simulink model "TosAeb"  (R2021a)
settings: Solver=FixedStepDiscrete  StartTime=0.0  StopTime=inf  FixedStep=0.01  RelTol=1e-3
undefined symbols (not defined in the file or in its folder): ACCModSts, AccSts1, AEB_ObjectMovingOrientation, AEB_ObjectMovingState, CN_BTCActive, CN_CurrentGearR, CN_CurrentGearR_DHT, CN_PTCActive, CN_VDCActive, CN_VSC_EspOff, CN_VSC_FCW_AEB_Error, CN_VSC_FCW_AEB_NoDispay, CN_VSC_FCW_AEB_PerfmDgdtn, CN_VSC_FCW_AEB_TempUnAvlbl, CN_VSC_VehStandstill, CNaccelAvoidMax, CNaccelAvoidMin, CNbrakePedalPress, CNcomfortStrLeftAccelMax, CNcomfortStrLeftAccelMin, CNcomfortStrRightAccelMax, CNcomfortStrRightAccelMin, CNDrvDoorOpen, CNDrvModeEco, CNDrvModeNorm, CNDrvModeOther, CNDrvModeSport, CNDrvSBRreminder, CNNull, CNobjInvalidID, CNPassDoorOpen, CNPassSBRreminder, CNPEBSAccelNegInf, CNPEBSAccelNull, CNPEBSTimeInf, CNPEBSTimeNull, CNSTOPMAX, CNSTOPNULL, CNsubjWidth, CNSwtOFF, CNSwtON, CNTTCMAX, CNTTCNULL, CNVehConfigAEBCycNone, CNVehConfigAEBNone, CNVehConfigAEBPedNone, CNVehConfigFCWNone, CNVehConfigJANone, CollisionSeverity, FALSE, FCWSnvty, JACycObjCalcInfoDefaultParams, JAPedObjCalcInfoDefaultParams, JAVehObjCalcInfoDefaultParams, latCycObjCalcInfoDefaultParams, latPedObjCalcInfoDefaultParams, longObjCalcInfoDefaultParams, ModSts, movingStatus, ObjectType ... (+1112)
libraries: simulink x3031, AEBSFunctionLib x50, Ta_Library x33, AEBSStateMachineLib x23, Mylibrary x12, simulink_extras x7, ObjSelect_lib x3, dspstat3 x2
(part 10 of 102)

== system TosAeb/FcwAebJa_Step_sys/ALG_AEBSystem/ALG_AEBController/AEB_VehicleStateCalcApp/VehSuppAborCondtionCalc ==
  InputSignal : Inport
  ABASuppAborConditonCalc : Subsystem
  AEBfullSuppAborConditonCalc : Subsystem
  AEBpartSuppAborConditonCalc : Subsystem
  AcuteWarningSuppAborConditonCalc : Subsystem
  Bus Creator : BusCreator  Inputs=16  OutDataTypeStr=Bus: BusVSCVehSuppAborCond
      settings: InheritFromInputs=off
  Bus Selector : BusSelector  OutputSignals=VSC_AEBFuncSwtSts.AEB_VehResp,VSC_FCW_AEB_FctSts.FCW_AEB_VehFctSts,vehMovingSts.vehUnstableSts,vehMovingSts.vehAssociatedSysStsSuppFlg,vehStateCalcSignalInput.vehConfigInfo.VehicleConfigAEB,vehStateCalcSignalInput.blockTime.blockTimeBrakeReady
  Bus Selector1 : BusSelector  OutputSignals=VSC_AEBFuncSwtSts.AEB_VehResp,VSC_FCW_AEB_FctSts.FCW_AEB_VehFctSts,vehMovingSts.vehUnstableSts,vehMovingSts.vehAssociatedSysStsSuppFlg,vehStateCalcSignalInput.vehConfigInfo.VehicleConfigAEB,vehStateCalcSignalInput.blockTime.blockTimeBrakeReady
  Bus Selector2 : BusSelector  OutputSignals=VSC_AEBFuncSwtSts.AEB_VehResp,VSC_FCW_AEB_FctSts.FCW_AEB_VehFctSts,vehMovingSts.vehUnstableSts,vehMovingSts.vehAssociatedSysStsSuppFlg,vehStateCalcSignalInput.vehConfigInfo.VehicleConfigFCW,vehStateCalcSignalInput.blockTime.blockTimeWarning
  Bus Selector3 : BusSelector  OutputSignals=VSC_AEBFuncSwtSts.AEB_VehResp,VSC_FCW_AEB_FctSts.FCW_AEB_VehFctSts,vehMovingSts.vehUnstableSts,vehMovingSts.vehAssociatedSysStsSuppFlg,vehStateCalcSignalInput.vehConfigInfo.VehicleConfigFCW,vehStateCalcSignalInput.blockTime.blockTimeWarning
  Bus Selector4 : BusSelector  OutputSignals=VSC_AEBFuncSwtSts.AEB_VehResp,VSC_FCW_AEB_FctSts.FCW_AEB_VehFctSts,vehMovingSts.vehUnstableSts,vehMovingSts.vehAssociatedSysStsSuppFlg,vehStateCalcSignalInput.vehConfigInfo.VehicleConfigAEB,vehStateCalcSignalInput.blockTime.blockTimeBrake
  Bus Selector5 : BusSelector  OutputSignals=VSC_AEBFuncSwtSts.AEB_VehResp,VSC_FCW_AEB_FctSts.FCW_AEB_VehFctSts,vehMovingSts.vehUnstableSts,vehMovingSts.vehAssociatedSysStsSuppFlg,vehStateCalcSignalInput.vehConfigInfo.VehicleConfigFCW,vehStateCalcSignalInput.blockTime.blockTimeWarning
  Bus Selector6 : BusSelector  OutputSignals=VSC_AEBFuncSwtSts.AEB_VehResp,VSC_FCW_AEB_FctSts.FCW_AEB_VehFctSts,vehMovingSts.vehUnstableSts,vehMovingSts.vehAssociatedSysStsSuppFlg,vehStateCalcSignalInput.vehConfigInfo.VehicleConfigAEB,vehStateCalcSignalInput.blockTime.blockTimeBrake
  Bus Selector7 : BusSelector  OutputSignals=VSC_AEBFuncSwtSts.AEB_VehResp,VSC_FCW_AEB_FctSts.FCW_AEB_VehFctSts,vehMovingSts.vehUnstableSts,vehMovingSts.vehAssociatedSysStsSuppFlg,vehStateCalcSignalInput.vehConfigInfo.VehicleConfigAEB,vehStateCalcSignalInput.blockTime.blockTimeBrake
  EBASuppAborConditonCalc : Subsystem
  LatentWarningSuppAborConditonCalc : Subsystem
  PreWarningSuppAborConditonCalc : Subsystem
  PrefillSuppAborConditonCalc : Subsystem
  VSCVehSuppAborCond : Outport  OutDataTypeStr=Bus: BusVSCVehSuppAborCond
  wires:
  PreWarningSuppAborConditonCalc/1(VSCvehPreWarningSuppCond) -> Bus Creator/1  "VSCvehPreWarningSuppCond"
  PreWarningSuppAborConditonCalc/2(VSCvehPreWarningAborCond) -> Bus Creator/2  "VSCvehPreWarningAborCond"
  EBASuppAborConditonCalc/2(VSCvehEBAAborCond) -> Bus Creator/16  "VSCvehEBAAborCond"
  EBASuppAborConditonCalc/1(VSCvehEBASuppCond) -> Bus Creator/15  "VSCvehEBASuppCond"
  AcuteWarningSuppAborConditonCalc/1(VSCvehAcuteWarningSuppCond) -> Bus Creator/3  "VSCvehAcuteWarningSuppCond"
  AcuteWarningSuppAborConditonCalc/2(VSCvehAcuteWarningAborCond) -> Bus Creator/4  "VSCvehAcuteWarningAborCond"
  AEBfullSuppAborConditonCalc/2(VSCvehAEBfullAborCond) -> Bus Creator/14  "VSCvehAEBfullAborCond"
  AEBfullSuppAborConditonCalc/1(VSCvehAEBfullSuppCond) -> Bus Creator/13  "VSCvehAEBfullSuppCond"
  LatentWarningSuppAborConditonCalc/1(VSCvehLatentWarningSuppCond) -> Bus Creator/5  "VSCvehLatentWarningSuppCond"
  LatentWarningSuppAborConditonCalc/2(VSCvehLatentWarningAborCond) -> Bus Creator/6  "VSCvehLatentWarningAborCond"
  AEBpartSuppAborConditonCalc/2(VSCvehAEBpartAborCond) -> Bus Creator/12  "VSCvehAEBpartAborCond"
  AEBpartSuppAborConditonCalc/1(VSCvehAEBpartSuppCond) -> Bus Creator/11  "VSCvehAEBpartSuppCond"
  PrefillSuppAborConditonCalc/1(VSCvehPrefillSuppCond) -> Bus Creator/7  "VSCvehPrefillSuppCond"
  PrefillSuppAborConditonCalc/2(VSCvehPrefillAborCond) -> Bus Creator/8  "VSCvehPrefillAborCond"
  ABASuppAborConditonCalc/2(VSCvehABAAborCond) -> Bus Creator/10  "VSCvehABAAborCond"
  ABASuppAborConditonCalc/1(VSCvehABASuppCond) -> Bus Creator/9  "VSCvehABASuppCond"
  Bus Creator/1 -> VSCVehSuppAborCond/1
  Bus Selector7/6 -> EBASuppAborConditonCalc/6(blockTimeBrake)  "<blockTimeBrake>"
  Bus Selector7/5 -> EBASuppAborConditonCalc/5("vehConfigAEB ")  "<VehicleConfigAEB>"
  Bus Selector7/4 -> EBASuppAborConditonCalc/4(vehAssociatedSysStateSuppCond)  "<vehAssociatedSysStsSuppFlg>"
  Bus Selector7/3 -> EBASuppAborConditonCalc/3(vehUnstableStateFlg)  "<vehUnstableSts>"
  Bus Selector7/2 -> EBASuppAborConditonCalc/2(FCW_AEB_VehFctSts)  "<FCW_AEB_VehFctSts>"
  Bus Selector7/1 -> EBASuppAborConditonCalc/1(AEB_VehResp)  "<AEB_VehResp>"
  Bus Selector6/6 -> AEBpartSuppAborConditonCalc/6(blockTimeBrake)  "<blockTimeBrake>"
  Bus Selector6/5 -> AEBpartSuppAborConditonCalc/5("vehConfigAEB ")  "<VehicleConfigAEB>"
  Bus Selector6/4 -> AEBpartSuppAborConditonCalc/4(vehAssociatedSysStateSuppCond)  "<vehAssociatedSysStsSuppFlg>"
  Bus Selector6/3 -> AEBpartSuppAborConditonCalc/3(vehUnstableStateFlg)  "<vehUnstableSts>"
  Bus Selector6/2 -> AEBpartSuppAborConditonCalc/2(FCW_AEB_VehFctSts)  "<FCW_AEB_VehFctSts>"
  Bus Selector6/1 -> AEBpartSuppAborConditonCalc/1(AEB_VehResp)  "<AEB_VehResp>"
  InputSignal/1 -> Bus Selector2/1, Bus Selector5/1, Bus Selector/1, Bus Selector1/1, Bus Selector6/1, Bus Selector4/1, Bus Selector7/1, Bus Selector3/1
  Bus Selector4/6 -> AEBfullSuppAborConditonCalc/6(blockTimeBrake)  "<blockTimeBrake>"
  Bus Selector4/5 -> AEBfullSuppAborConditonCalc/5("vehConfigAEB ")  "<VehicleConfigAEB>"
  Bus Selector4/4 -> AEBfullSuppAborConditonCalc/4(vehAssociatedSysStateSuppCond)  "<vehAssociatedSysStsSuppFlg>"
  Bus Selector4/3 -> AEBfullSuppAborConditonCalc/3(vehUnstableStateFlg)  "<vehUnstableSts>"
  Bus Selector4/2 -> AEBfullSuppAborConditonCalc/2(FCW_AEB_VehFctSts)  "<FCW_AEB_VehFctSts>"
  Bus Selector4/1 -> AEBfullSuppAborConditonCalc/1(VSCvehAEB_VehResp)  "<AEB_VehResp>"
  Bus Selector5/6 -> LatentWarningSuppAborConditonCalc/6(blockTimeWarning)  "<blockTimeWarning>"
  Bus Selector5/5 -> LatentWarningSuppAborConditonCalc/5("vehConfigFCW ")  "<VehicleConfigFCW>"
  Bus Selector5/4 -> LatentWarningSuppAborConditonCalc/4(vehAssociatedSysStateSuppCond)  "<vehAssociatedSysStsSuppFlg>"
  Bus Selector5/3 -> LatentWarningSuppAborConditonCalc/3(vehUnstableStateFlg)  "<vehUnstableSts>"
  Bus Selector5/2 -> LatentWarningSuppAborConditonCalc/2(FCW_AEB_VehFctSts)  "<FCW_AEB_VehFctSts>"
  Bus Selector5/1 -> LatentWarningSuppAborConditonCalc/1(AEB_VehResp)  "<AEB_VehResp>"
  Bus Selector3/6 -> AcuteWarningSuppAborConditonCalc/6(blockTimeWarning)  "<blockTimeWarning>"
  Bus Selector3/5 -> AcuteWarningSuppAborConditonCalc/5("vehConfigFCW ")  "<VehicleConfigFCW>"
  Bus Selector3/4 -> AcuteWarningSuppAborConditonCalc/4(vehAssociatedSysStateSuppCond)  "<vehAssociatedSysStsSuppFlg>"
  Bus Selector3/3 -> AcuteWarningSuppAborConditonCalc/3(vehUnstableStateFlg)  "<vehUnstableSts>"
  Bus Selector3/2 -> AcuteWarningSuppAborConditonCalc/2(FCW_AEB_VehFctSts)  "<FCW_AEB_VehFctSts>"
  Bus Selector3/1 -> AcuteWarningSuppAborConditonCalc/1(AEB_VehResp)  "<AEB_VehResp>"
  Bus Selector2/6 -> PreWarningSuppAborConditonCalc/6(blockTimeWarning)  "<blockTimeWarning>"
  Bus Selector2/5 -> PreWarningSuppAborConditonCalc/5("vehConfigFCW ")  "<VehicleConfigFCW>"
  Bus Selector2/4 -> PreWarningSuppAborConditonCalc/4(vehAssociatedSysSuppStateFlg)  "<vehAssociatedSysStsSuppFlg>"
  Bus Selector2/3 -> PreWarningSuppAborConditonCalc/3(vehUnstableStateFlg)  "<vehUnstableSts>"
  Bus Selector2/2 -> PreWarningSuppAborConditonCalc/2(FCW_AEB_VehFctSts)  "<FCW_AEB_VehFctSts>"
  Bus Selector2/1 -> PreWarningSuppAborConditonCalc/1(AEB_VehResp)  "<AEB_VehResp>"
  Bus Selector1/6 -> ABASuppAborConditonCalc/6(blockTimeBrakeReady)  "<blockTimeBrakeReady>"
  Bus Selector1/5 -> ABASuppAborConditonCalc/5("vehConfigAEB ")  "<VehicleConfigAEB>"
  Bus Selector1/4 -> ABASuppAborConditonCalc/4(vehAssociatedSysStateSuppCond)  "<vehAssociatedSysStsSuppFlg>"
  Bus Selector1/3 -> ABASuppAborConditonCalc/3(vehUnstableStateFlg)  "<vehUnstableSts>"
  Bus Selector1/2 -> ABASuppAborConditonCalc/2(FCW_AEB_VehFctSts)  "<FCW_AEB_VehFctSts>"
  Bus Selector1/1 -> ABASuppAborConditonCalc/1(AEB_VehResp)  "<AEB_VehResp>"
  Bus Selector/6 -> PrefillSuppAborConditonCalc/6(blockTimeBrakeReady)  "<blockTimeBrakeReady>"
  Bus Selector/1 -> PrefillSuppAborConditonCalc/1(AEB_VehResp)  "<AEB_VehResp>"
  Bus Selector/5 -> PrefillSuppAborConditonCalc/5("vehConfigAEB ")  "<VehicleConfigAEB>"
  Bus Selector/2 -> PrefillSuppAborConditonCalc/2(FCW_AEB_VehFctSts)  "<FCW_AEB_VehFctSts>"
  Bus Selector/4 -> PrefillSuppAborConditonCalc/4(vehAssociatedSysStateSuppCond)  "<vehAssociatedSysStsSuppFlg>"
  Bus Selector/3 -> PrefillSuppAborConditonCalc/3(vehUnstableStateFlg)  "<vehUnstableSts>"

== system TosAeb/FcwAebJa_Step_sys/ALG_AEBSystem/ALG_AEBController/AEB_VehicleStateCalcApp/vehStandStillCalc ==
  note: FLWheelSpd + FRWheelSpd + RLWheelSpd + RRWheelSpd
      | subjWheelSpd = ---------------------------------------------------------------------------------------
      | 											4
  FLWheelSpd : Inport
  FRWheelSpd : Inport  Port=2
  RLWheelSpd : Inport  Port=3
  RRWheelSpd : Inport  Port=4
  vehicleStandstill : Inport  Port=5
  Add : Sum  Inputs=4
  Compare To Constant : simulink/Logic and Bit Operations/Compare To Constant  relop===  const=CN_VSC_VehStandstill  OutDataTypeStr=boolean
  Compare To Constant1 : simulink/Logic and Bit Operations/Compare To Constant  relop=<  const=P_AEB_vehStateCalc_VehWheelThres  OutDataTypeStr=boolean
  Gain : Gain  Gain=0.25
  Logical Operator : Logic  Operator=OR  AllPortsSameDT=off  OutDataTypeStr=boolean
  VSCVehStandStill : Outport
  wires:
  Logical Operator/1 -> VSCVehStandStill/1
  Compare To Constant/1 -> Logical Operator/2
  Compare To Constant1/1 -> Logical Operator/1
  vehicleStandstill/1 -> Compare To Constant/1
  Gain/1 -> Compare To Constant1/1  "subjWheelSpdAverage"
  Add/1 -> Gain/1
  RRWheelSpd/1 -> Add/4
  RLWheelSpd/1 -> Add/3
  FRWheelSpd/1 -> Add/2
  FLWheelSpd/1 -> Add/1

== system TosAeb/FcwAebJa_Step_sys/ALG_AEBSystem/ALG_AEBInput/JaObjInfoMap/JaObjInfoMap ==
  TOS_AEB_JAOBJ : Inport  OutDataTypeStr=Bus: Object_Struct
  Bus Creator : BusCreator  Inputs=54  OutDataTypeStr=Bus: BusAEBJAObjBus  NonVirtualBus=on
      settings: InheritFromInputs=off
  Bus Selector2 : BusSelector  OutputSignals=objID,objType,objLength,objWidth,objHeight,azimuthAngle,yawAngle,movingStatus,movingDirection,dxCenter,dyCenter,vxRel,vyRel,axRel,ayRel,dxCenterStd,dyCenterStd,vxRelStd,vyRelStd,axRelStd,ayRelStd,objLengthStd,objWidthStd,yawAngleStd,objExitProb,objID,objType,movingStatus,movingDirection,dxCenter,dyCenter,vxRel,vyRel,objID,objType,movingStatus,movingDirection,dxCenter,dyCenter,vxRel,vyRel
  Data Type Conversion{,2..8,11..30,32..36,38..46,48..54} (49 alike) : DataTypeConversion
  Data Type Conversion1 : DataTypeConversion  OutDataTypeStr=Enum: AEB_ObjectMovingState
  Data Type Conversion10 : DataTypeConversion  OutDataTypeStr=uint16
  Data Type Conversion31 : DataTypeConversion  OutDataTypeStr=Enum: ObjectTypeCopy
  Data Type Conversion47 : DataTypeConversion  OutDataTypeStr=uint16
  Data Type Conversion55 : DataTypeConversion  OutDataTypeStr=uint16
  FusionType : Constant  OutDataTypeStr=single
  MovingStatus_Subsystem : Subsystem
  ObjType_Subsystem : Subsystem
  ObjectTypeCameraConfirmed : Constant  OutDataTypeStr=single
  Subsystem : Subsystem
  Subsystem1 : Subsystem
  dxFirstDetection : Constant  OutDataTypeStr=single
  dyFirstDetection : Constant  OutDataTypeStr=single
  dz : Constant  OutDataTypeStr=single
  objUpdate : Constant  Value=0  OutDataTypeStr=single
  objectIsObstacleProb : Constant  OutDataTypeStr=single
  objectLifeCycle : Constant  Value=0  OutDataTypeStr=single
  objectMeasurementStatus : Constant  Value=0  OutDataTypeStr=single
  objectMovingOrientationProb : Constant  OutDataTypeStr=single
  objectMovingStateProb : Constant  OutDataTypeStr=single
  objectRCS : Constant  OutDataTypeStr=single
  objectTypeProb : Constant  OutDataTypeStr=single
  JaObjInfo : Outport  OutDataTypeStr=Bus: BusAEBJAObjBus
  wires:
  ObjType_Subsystem/1(ObjType) -> Data Type Conversion31/1  "ObjectType"
  objectMeasurementStatus/1 -> Data Type Conversion35/1
  Bus Selector2/17 -> Data Type Conversion19/1  "<dyCenterStd>"
  objectTypeProb/1 -> Data Type Conversion24/1
  dz/1 -> Data Type Conversion18/1
  Bus Selector2/21 -> Data Type Conversion12/1  "<ayRelStd>"
  Bus Selector2/13 -> Data Type Conversion29/1  "<vyRel>"
  Bus Selector2/25 -> Data Type Conversion44/1  "<objExitProb>"
  Bus Selector2/18 -> Data Type Conversion23/1  "<vxRelStd>"
  objectLifeCycle/1 -> Data Type Conversion34/1
  objectRCS/1 -> Data Type Conversion36/1
  dyFirstDetection/1 -> Data Type Conversion6/1
  Bus Selector2/10 -> Data Type Conversion5/1  "<dxCenter>"
  Bus Selector2/14 -> Data Type Conversion28/1  "<axRel>"
  Bus Selector2/22 -> Data Type Conversion27/1  "<objLengthStd>"
  Bus Selector2/7 -> Data Type Conversion2/1  "<yawAngle>"
  Bus Selector2/3 -> Data Type Conversion15/1  "<objLength>"
  Bus Selector2/4 -> Data Type Conversion14/1  "<objWidth>"
  Bus Selector2/32 -> Data Type Conversion39/1  "<vxRel>"
  Bus Selector2/28 -> Data Type Conversion42/1  "<movingStatus>"
  Bus Selector2/36 -> Data Type Conversion52/1  "<movingStatus>"
  Bus Selector2/40 -> Data Type Conversion49/1  "<vxRel>"
  Bus Selector2/33 -> Data Type Conversion45/1  "<vyRel>"
  Bus Selector2/41 -> Data Type Conversion53/1  "<vyRel>"
  Bus Selector2/37 -> Data Type Conversion51/1  "<movingDirection>"
  Bus Selector2/29 -> Data Type Conversion41/1  "<movingDirection>"
  objUpdate/1 -> Data Type Conversion30/1
  Bus Selector2/20 -> Data Type Conversion16/1  "<axRelStd>"
  objectMovingOrientationProb/1 -> Data Type Conversion32/1
  Bus Selector2/12 -> Data Type Conversion17/1  "<vxRel>"
  Bus Selector2/16 -> Data Type Conversion20/1  "<dxCenterStd>"
  Bus Selector2/24 -> Data Type Conversion25/1  "<yawAngleStd>"
  FusionType/1 -> Data Type Conversion11/1
  Bus Selector2/2 -> ObjType_Subsystem/1(Alg_ObjType)  "<objType>"
  Bus Selector2/6 -> Data Type Conversion3/1  "<azimuthAngle>"
  objectIsObstacleProb/1 -> Data Type Conversion43/1
  Bus Selector2/15 -> Data Type Conversion21/1  "<ayRel>"
  objectMovingStateProb/1 -> Data Type Conversion33/1
  Bus Selector2/11 -> Data Type Conversion4/1  "<dyCenter>"
  Bus Selector2/19 -> Data Type Conversion22/1  "<vyRelStd>"
  dxFirstDetection/1 -> Data Type Conversion7/1
  Bus Selector2/23 -> Data Type Conversion26/1  "<objWidthStd>"
  Bus Selector2/9 -> Data Type Conversion/1  "<movingDirection>"
  Bus Selector2/1 -> Data Type Conversion10/1  "<objID>"
  Bus Selector2/5 -> Data Type Conversion13/1  "<objHeight>"
  Bus Selector2/38 -> Data Type Conversion50/1  "<dxCenter>"
  Bus Selector2/34 -> Data Type Conversion55/1  "<objID>"
  Bus Selector2/30 -> Data Type Conversion40/1  "<dxCenter>"
  Bus Selector2/26 -> Data Type Conversion47/1  "<objID>"
  Bus Selector2/39 -> Data Type Conversion48/1  "<dyCenter>"
  Bus Selector2/31 -> Data Type Conversion38/1  "<dyCenter>"
  Bus Selector2/27 -> Data Type Conversion46/1  "<objType>"
  Bus Selector2/35 -> Data Type Conversion54/1  "<objType>"
  TOS_AEB_JAOBJ/1 -> Bus Selector2/1
  Data Type Conversion19/1 -> Bus Creator/22  "dyVariance"
  ObjectTypeCameraConfirmed/1 -> Data Type Conversion8/1
  Data Type Conversion47/1 -> Bus Creator/39  "FR_ObjectID"
  Data Type Conversion45/1 -> Bus Creator/46  "FR_Vy"
  Data Type Conversion55/1 -> Bus Creator/47  "FC_ObjectID"
  Data Type Conversion54/1 -> Subsystem1/1(FC_ObjectType)  "FC_ObjectType"
  Data Type Conversion38/1 -> Bus Creator/44  "FR_Dy"
  Data Type Conversion39/1 -> Bus Creator/45  "FR_Vx"
  Subsystem/1(FR_ObjectTypeFilt) -> Bus Creator/40  "FR_ObjectType"
  Data Type Conversion41/1 -> Subsystem/3(FR_ObjectMovingOrientation)  "FR_ObjectMovingOrientation"
  Subsystem1/3(FC_ObjectMovingOrientationFilt) -> Bus Creator/50  "FC_ObjectMovingOrientation"
  Subsystem1/2(FC_ObjectMovingStateFilt) -> Bus Creator/49  "FC_ObjectMovingState"
  Data Type Conversion52/1 -> Subsystem1/2(FC_ObjectMovingState)  "FC_ObjectMovingState"
  Data Type Conversion48/1 -> Bus Creator/52  "FC_Dy"
  Subsystem/3(FR_ObjectMovingOrientationFilt) -> Bus Creator/42  "FR_ObjectMovingOrientation"
  Subsystem/2(FR_ObjectMovingStateFilt) -> Bus Creator/41  "FR_ObjectMovingState"
  Data Type Conversion49/1 -> Bus Creator/53  "FC_Vx"
  Data Type Conversion51/1 -> Subsystem1/3(FC_ObjectMovingOrientation)  "FC_ObjectMovingOrientation"
  Data Type Conversion42/1 -> Subsystem/2(FR_ObjectMovingState)  "FR_ObjectMovingState"
  Data Type Conversion50/1 -> Bus Creator/51  "FC_Dx"
  Data Type Conversion53/1 -> Bus Creator/54  "FC_Vy"
  Data Type Conversion46/1 -> Subsystem/1(FR_ObjectType)  "FR_ObjectType"
  Subsystem1/1(FC_ObjectTypeFilt) -> Bus Creator/48  "FC_ObjectType"
  Data Type Conversion40/1 -> Bus Creator/43  "FR_Dx"
  Data Type Conversion17/1 -> Bus Creator/17  "vx"
  Bus Creator/1 -> JaObjInfo/1
  Data Type Conversion/1 -> Bus Creator/11  "ObjectMovingOrientation"
  Data Type Conversion1/1 -> Bus Creator/10  "ObjectMovingState"
  Data Type Conversion2/1 -> Bus Creator/9  "ObjectYawAngle"
  Data Type Conversion3/1 -> Bus Creator/8  "ObjectAzimuthAngle"
  Data Type Conversion4/1 -> Bus Creator/15  "dy"
  Data Type Conversion5/1 -> Bus Creator/14  "dx"
  Data Type Conversion6/1 -> Bus Creator/13  "dyFisrtDetection"
  Data Type Conversion7/1 -> Bus Creator/12  "dxFisrtDetection"
  Data Type Conversion8/1 -> Bus Creator/4  "ObjectTypeCameraConfirmed"
  Data Type Conversion13/1 -> Bus Creator/7  "ObjectHight"
  Data Type Conversion11/1 -> Bus Creator/1  "FusionType"
  Data Type Conversion31/1 -> Bus Creator/3  "ObjectType"
  Data Type Conversion10/1 -> Bus Creator/2  "ObjectID"
  Data Type Conversion14/1 -> Bus Creator/6  "ObjectWidth"
  Data Type Conversion15/1 -> Bus Creator/5  "ObjectLength"
  Data Type Conversion12/1 -> Bus Creator/26  "ayVariance"
  Data Type Conversion16/1 -> Bus Creator/25  "axVariance"
  Data Type Conversion18/1 -> Bus Creator/16  "dz"
  Data Type Conversion20/1 -> Bus Creator/21  "dxVariance"
  Data Type Conversion21/1 -> Bus Creator/20  "ay"
  Data Type Conversion22/1 -> Bus Creator/24  "vyVariance"
  Data Type Conversion24/1 -> Bus Creator/30  "ObjectTypeProb"
  Data Type Conversion25/1 -> Bus Creator/29  "ObjectYawAngleVar"
  Data Type Conversion23/1 -> Bus Creator/23  "vxVariance"
  Data Type Conversion27/1 -> Bus Creator/27  "ObjectLengthVar"
  Data Type Conversion26/1 -> Bus Creator/28  "ObjectWidthVar"
  Data Type Conversion28/1 -> Bus Creator/19  "ax"
  Data Type Conversion29/1 -> Bus Creator/18  "vy"
  Data Type Conversion32/1 -> Bus Creator/32  "ObjectMovingOrientationProb"
  Data Type Conversion34/1 -> Bus Creator/37  "ObjectLifeCycle"
  Data Type Conversion33/1 -> Bus Creator/31  "ObjectMovingStateProb"
  Data Type Conversion35/1 -> Bus Creator/36  "ObjectMeasurementStatus"
  Data Type Conversion36/1 -> Bus Creator/35  "ObjectRCS"
  Data Type Conversion43/1 -> Bus Creator/34  "ObjectIsObstacleProb"
  Data Type Conversion44/1 -> Bus Creator/33  "ObjectExistProb"
  Data Type Conversion30/1 -> Bus Creator/38  "ObjUpdate"
  MovingStatus_Subsystem/1(MovingStatusOut) -> Data Type Conversion1/1
  Bus Selector2/8 -> MovingStatus_Subsystem/1(Alg_MovingStatus)  "<movingStatus>"

== system TosAeb/FcwAebJa_Step_sys/ALG_AEBSystem/ALG_AEBInput/LatObjInfoMap/latObjInfoMap ==
  TOS_AEB_LATOBJ : Inport  OutDataTypeStr=Bus: Object_Struct
  Bus Creator : BusCreator  Inputs=54  OutDataTypeStr=Bus: BusAEBLatObj  NonVirtualBus=on
      settings: InheritFromInputs=off
  Bus Selector2 : BusSelector  OutputSignals=objID,objType,objLength,objWidth,objHeight,azimuthAngle,yawAngle,movingStatus,movingDirection,dxCenter,dyCenter,vxRel,vyRel,axRel,ayRel,dxCenterStd,dyCenterStd,vxRelStd,vyRelStd,axRelStd,ayRelStd,objLengthStd,objWidthStd,yawAngleStd,objExitProb,objID,objType,movingStatus,movingDirection,dxCenter,dyCenter,vxRel,vyRel,objID,objType,movingStatus,movingDirection,dxCenter,dyCenter,vxRel,vyRel
  Data Type Conversion{,2..8,11..30,32..36,38..46,48..54} (49 alike) : DataTypeConversion
  Data Type Conversion10 : DataTypeConversion  OutDataTypeStr=uint16
  Data Type Conversion31 : DataTypeConversion  OutDataTypeStr=Enum: ObjectTypeCopy
  Data Type Conversion47 : DataTypeConversion  OutDataTypeStr=uint16
  Data Type Conversion55 : DataTypeConversion  OutDataTypeStr=uint16
  Data Type Conversion9 : DataTypeConversion  OutDataTypeStr=Enum: AEB_ObjectMovingState
  FusionType : Constant  OutDataTypeStr=single
  MovingStatus_Subsystem : Subsystem
  ObjType_Subsystem : Subsystem
  ObjectTypeCameraConfirmed : Constant  OutDataTypeStr=single
  Subsystem : Subsystem
  Subsystem1 : Subsystem
  dxFirstDetection : Constant  OutDataTypeStr=single
  dyFirstDetection : Constant  OutDataTypeStr=single
  dz : Constant  OutDataTypeStr=single
  objUpdate : Constant  Value=0  OutDataTypeStr=single
  objectIsObstacleProb : Constant  OutDataTypeStr=single
  objectLifeCycle : Constant  Value=0  OutDataTypeStr=single
  objectMeasurementStatus : Constant  Value=0  OutDataTypeStr=single
  objectMovingOrientationProb : Constant  OutDataTypeStr=single
  objectMovingStateProb : Constant  OutDataTypeStr=single
  objectRCS : Constant  OutDataTypeStr=single
  objectTypeProb : Constant  OutDataTypeStr=single
  latObjInfo : Outport  OutDataTypeStr=Bus: BusAEBLatObj
  wires:
  Data Type Conversion44/1 -> Bus Creator/33  "ObjectExistProb"
  Data Type Conversion43/1 -> Bus Creator/34  "ObjectIsObstacleProb"
  Data Type Conversion36/1 -> Bus Creator/35  "ObjectRCS"
  Data Type Conversion35/1 -> Bus Creator/36  "ObjectMeasurementStatus"
  Data Type Conversion33/1 -> Bus Creator/31  "ObjectMovingStateProb"
  Data Type Conversion34/1 -> Bus Creator/37  "ObjectLifeCycle"
  Data Type Conversion32/1 -> Bus Creator/32  "ObjectMovingOrientationProb"
  Data Type Conversion29/1 -> Bus Creator/18  "vy"
  Data Type Conversion28/1 -> Bus Creator/19  "ax"
  Data Type Conversion26/1 -> Bus Creator/28  "ObjectWidthVar"
  Data Type Conversion27/1 -> Bus Creator/27  "ObjectLengthVar"
  Data Type Conversion23/1 -> Bus Creator/23  "vxVariance"
  Data Type Conversion25/1 -> Bus Creator/29  "ObjectYawAngleVar"
  Data Type Conversion24/1 -> Bus Creator/30  "ObjectTypeProb"
  Data Type Conversion22/1 -> Bus Creator/24  "vyVariance"
  Data Type Conversion21/1 -> Bus Creator/20  "ay"
  Data Type Conversion20/1 -> Bus Creator/21  "dxVariance"
  Data Type Conversion19/1 -> Bus Creator/22  "dyVariance"
  Data Type Conversion18/1 -> Bus Creator/16  "dz"
  Data Type Conversion16/1 -> Bus Creator/25  "axVariance"
  Data Type Conversion12/1 -> Bus Creator/26  "ayVariance"
  Data Type Conversion17/1 -> Bus Creator/17  "vx"
  Data Type Conversion15/1 -> Bus Creator/5  "ObjectLength"
  Data Type Conversion14/1 -> Bus Creator/6  "ObjectWidth"
  Data Type Conversion10/1 -> Bus Creator/2  "ObjectID"
  Data Type Conversion31/1 -> Bus Creator/3  "ObjectType"
  Data Type Conversion11/1 -> Bus Creator/1  "FusionType"
  Data Type Conversion13/1 -> Bus Creator/7  "ObjectHight"
  Data Type Conversion8/1 -> Bus Creator/4  "ObjectTypeCameraConfirmed"
  Data Type Conversion7/1 -> Bus Creator/12  "dxFisrtDetection"
  Data Type Conversion6/1 -> Bus Creator/13  "dyFisrtDetection"
  Data Type Conversion5/1 -> Bus Creator/14  "dx"
  Data Type Conversion4/1 -> Bus Creator/15  "dy"
  Data Type Conversion3/1 -> Bus Creator/8  "ObjectAzimuthAngle"
  Data Type Conversion2/1 -> Bus Creator/9  "ObjectYawAngle"
  Data Type Conversion9/1 -> Bus Creator/10  "ObjectMovingState"
  Data Type Conversion/1 -> Bus Creator/11  "ObjectMovingOrientation"
  Bus Selector2/25 -> Data Type Conversion44/1  "<objExitProb>"
  Bus Selector2/1 -> Data Type Conversion10/1  "<objID>"
  Bus Selector2/24 -> Data Type Conversion25/1  "<yawAngleStd>"
  Bus Selector2/2 -> ObjType_Subsystem/1(Alg_ObjType)  "<objType>"
  Bus Selector2/23 -> Data Type Conversion26/1  "<objWidthStd>"
  Bus Selector2/22 -> Data Type Conversion27/1  "<objLengthStd>"
  Bus Selector2/3 -> Data Type Conversion15/1  "<objLength>"
  Bus Selector2/21 -> Data Type Conversion12/1  "<ayRelStd>"
  Bus Selector2/4 -> Data Type Conversion14/1  "<objWidth>"
  Bus Selector2/20 -> Data Type Conversion16/1  "<axRelStd>"
  Bus Selector2/5 -> Data Type Conversion13/1  "<objHeight>"
  Bus Selector2/19 -> Data Type Conversion22/1  "<vyRelStd>"
  Bus Selector2/6 -> Data Type Conversion3/1  "<azimuthAngle>"
  Bus Selector2/18 -> Data Type Conversion23/1  "<vxRelStd>"
  Bus Selector2/7 -> Data Type Conversion2/1  "<yawAngle>"
  Bus Selector2/17 -> Data Type Conversion19/1  "<dyCenterStd>"
  Bus Selector2/16 -> Data Type Conversion20/1  "<dxCenterStd>"
  Bus Selector2/9 -> Data Type Conversion/1  "<movingDirection>"
  Bus Selector2/15 -> Data Type Conversion21/1  "<ayRel>"
  Bus Selector2/14 -> Data Type Conversion28/1  "<axRel>"
  Bus Selector2/13 -> Data Type Conversion29/1  "<vyRel>"
  Bus Selector2/10 -> Data Type Conversion5/1  "<dxCenter>"
  Bus Selector2/12 -> Data Type Conversion17/1  "<vxRel>"
  Bus Selector2/11 -> Data Type Conversion4/1  "<dyCenter>"
  Bus Creator/1 -> latObjInfo/1
  Data Type Conversion30/1 -> Bus Creator/38  "ObjUpdate"
  Data Type Conversion52/1 -> Subsystem1/2(FC_ObjectMovingState)  "FC_ObjectMovingState"
  Data Type Conversion48/1 -> Bus Creator/52  "FC_Dy"
  Data Type Conversion50/1 -> Bus Creator/51  "FC_Dx"
  Data Type Conversion51/1 -> Subsystem1/3(FC_ObjectMovingOrientation)  "FC_ObjectMovingOrientation"
  Data Type Conversion54/1 -> Subsystem1/1(FC_ObjectType)  "FC_ObjectType"
  Data Type Conversion46/1 -> Subsystem/1(FR_ObjectType)  "FR_ObjectType"
  Subsystem1/3(FC_ObjectMovingOrientationFilt) -> Bus Creator/50  "FC_ObjectMovingOrientation"
  Subsystem/3(FR_ObjectMovingOrientationFilt) -> Bus Creator/42  "FR_ObjectMovingOrientation"
  Bus Selector2/27 -> Data Type Conversion46/1  "<objType>"
  Subsystem1/1(FC_ObjectTypeFilt) -> Bus Creator/48  "FC_ObjectType"
  Data Type Conversion41/1 -> Subsystem/3(FR_ObjectMovingOrientation)  "FR_ObjectMovingOrientation"
  Subsystem1/2(FC_ObjectMovingStateFilt) -> Bus Creator/49  "FC_ObjectMovingState"
  Subsystem/1(FR_ObjectTypeFilt) -> Bus Creator/40  "FR_ObjectType"
  Bus Selector2/34 -> Data Type Conversion55/1  "<objID>"
  Data Type Conversion53/1 -> Bus Creator/54  "FC_Vy"
  Subsystem/2(FR_ObjectMovingStateFilt) -> Bus Creator/41  "FR_ObjectMovingState"
  Data Type Conversion49/1 -> Bus Creator/53  "FC_Vx"
  Data Type Conversion55/1 -> Bus Creator/47  "FC_ObjectID"
  Data Type Conversion42/1 -> Subsystem/2(FR_ObjectMovingState)  "FR_ObjectMovingState"
  Bus Selector2/26 -> Data Type Conversion47/1  "<objID>"
  Bus Selector2/40 -> Data Type Conversion49/1  "<vxRel>"
  Data Type Conversion45/1 -> Bus Creator/46  "FR_Vy"
  Bus Selector2/36 -> Data Type Conversion52/1  "<movingStatus>"
  Bus Selector2/39 -> Data Type Conversion48/1  "<dyCenter>"
  Data Type Conversion39/1 -> Bus Creator/45  "FR_Vx"
  Bus Selector2/32 -> Data Type Conversion39/1  "<vxRel>"
  Bus Selector2/35 -> Data Type Conversion54/1  "<objType>"
  Data Type Conversion38/1 -> Bus Creator/44  "FR_Dy"
  Data Type Conversion40/1 -> Bus Creator/43  "FR_Dx"
  Bus Selector2/38 -> Data Type Conversion50/1  "<dxCenter>"
  Data Type Conversion47/1 -> Bus Creator/39  "FR_ObjectID"
  Bus Selector2/30 -> Data Type Conversion40/1  "<dxCenter>"
  Bus Selector2/41 -> Data Type Conversion53/1  "<vyRel>"
  Bus Selector2/37 -> Data Type Conversion51/1  "<movingDirection>"
  Bus Selector2/29 -> Data Type Conversion41/1  "<movingDirection>"
  Bus Selector2/28 -> Data Type Conversion42/1  "<movingStatus>"
  Bus Selector2/31 -> Data Type Conversion38/1  "<dyCenter>"
  Bus Selector2/33 -> Data Type Conversion45/1  "<vyRel>"
  TOS_AEB_LATOBJ/1 -> Bus Selector2/1
  FusionType/1 -> Data Type Conversion11/1
  ObjectTypeCameraConfirmed/1 -> Data Type Conversion8/1
  dxFirstDetection/1 -> Data Type Conversion7/1
  dyFirstDetection/1 -> Data Type Conversion6/1
  dz/1 -> Data Type Conversion18/1
  objectTypeProb/1 -> Data Type Conversion24/1
  objectMovingStateProb/1 -> Data Type Conversion33/1
  objectMovingOrientationProb/1 -> Data Type Conversion32/1
  objectIsObstacleProb/1 -> Data Type Conversion43/1
  objectRCS/1 -> Data Type Conversion36/1
  objectMeasurementStatus/1 -> Data Type Conversion35/1
  objectLifeCycle/1 -> Data Type Conversion34/1
  objUpdate/1 -> Data Type Conversion30/1
  ObjType_Subsystem/1(ObjType) -> Data Type Conversion31/1  "ObjectType"
  MovingStatus_Subsystem/1(MovingStatusOut) -> Data Type Conversion9/1
  Bus Selector2/8 -> MovingStatus_Subsystem/1(Alg_MovingStatus)  "<movingStatus>"

== system TosAeb/FcwAebJa_Step_sys/ALG_AEBSystem/ALG_AEBInput/LongObjInfoMap/MovingStatus_Subsystem ==
  Alg_MovingStatus : Inport
  InputMovingStatus : Subsystem  TreatAsAtomicUnit=on
  MovingStatusSwitch : Stateflow chart  TreatAsAtomicUnit=on
  MovingStatusOut : Outport
  wires:
  Alg_MovingStatus/1 -> InputMovingStatus/1(MovingStatus)
  MovingStatusSwitch/1(MovingStatus) -> MovingStatusOut/1
  InputMovingStatus/1(Input_Unknown) -> MovingStatusSwitch/1(Input_Unknown)
  InputMovingStatus/2(Input_Stationary) -> MovingStatusSwitch/2(Input_Stationary)
  InputMovingStatus/3(Input_Stopped) -> MovingStatusSwitch/3(Input_Stopped)
  InputMovingStatus/4(Input_Moving) -> MovingStatusSwitch/4(Input_Moving)

== system TosAeb/FcwAebJa_Step_sys/ALG_AEBSystem/ALG_AEBInput/LongObjInfoMap/ObjType_Subsystem ==
  Alg_ObjType : Inport
  InputObjectType : Subsystem  TreatAsAtomicUnit=on
  ObjectType : Stateflow chart  TreatAsAtomicUnit=on
  ObjType : Outport
  wires:
  InputObjectType/4(Input_ObjMotor) -> ObjectType/4(ObjMotor)
  InputObjectType/6(Input_ObjGeneral) -> ObjectType/6(ObjGeneral)
  InputObjectType/3(Input_ObjPed) -> ObjectType/3(ObjPed)
  InputObjectType/7(Input_Unknown) -> ObjectType/7(Unknown)
  InputObjectType/5(Input_ObjBicycle) -> ObjectType/5(ObjBicycle)
  InputObjectType/2(Input_ObjTruck) -> ObjectType/2(ObjTruck)
  InputObjectType/1(Input_ObjCar) -> ObjectType/1(ObjCar)
  ObjectType/1(ObjType) -> ObjType/1
  Alg_ObjType/1 -> InputObjectType/1(InputObjType)

== system TosAeb/FcwAebJa_Step_sys/ALG_AEBSystem/ALG_AEBInput/LongObjInfoMap/Subsystem ==
  FR_ObjectType : Inport
  FR_ObjectMovingState : Inport  Port=2
  FR_ObjectMovingOrientation : Inport  Port=3
  Data Type Conversion : DataTypeConversion  OutDataTypeStr=Enum: AEB_ObjectMovingOrientation
  Data Type Conversion1 : DataTypeConversion  OutDataTypeStr=Enum: ObjectTypeCopy
  Data Type Conversion2 : DataTypeConversion  OutDataTypeStr=Enum: AEB_ObjectMovingState
  MovingStatus_Subsystem : Subsystem
  ObjType_Subsystem : Subsystem
  FR_ObjectTypeFilt : Outport
  FR_ObjectMovingStateFilt : Outport  Port=2
  FR_ObjectMovingOrientationFilt : Outport  Port=3
  wires:
  Data Type Conversion2/1 -> FR_ObjectMovingStateFilt/1
  FR_ObjectMovingState/1 -> MovingStatus_Subsystem/1(Alg_MovingStatus)
  FR_ObjectType/1 -> ObjType_Subsystem/1(Alg_ObjType)
  ObjType_Subsystem/1(ObjType) -> Data Type Conversion1/1  "ObjectType"
  Data Type Conversion1/1 -> FR_ObjectTypeFilt/1  "ObjectType"
  MovingStatus_Subsystem/1(MovingStatusOut) -> Data Type Conversion2/1
  FR_ObjectMovingOrientation/1 -> Data Type Conversion/1
  Data Type Conversion/1 -> FR_ObjectMovingOrientationFilt/1  "ObjectMovingOrientation"

== system TosAeb/FcwAebJa_Step_sys/ALG_AEBSystem/ALG_AEBInput/LongObjInfoMap/Subsystem1 ==
  FC_ObjectType : Inport
  FC_ObjectMovingState : Inport  Port=2
  FC_ObjectMovingOrientation : Inport  Port=3
  Data Type Conversion : DataTypeConversion  OutDataTypeStr=Enum: AEB_ObjectMovingOrientation
  Data Type Conversion1 : DataTypeConversion  OutDataTypeStr=Enum: ObjectTypeCopy
  Enumerated Constant13 : simulink/Sources/Enumerated Constant  OutDataTypeStr=Enum: AEB_ObjectMovingState  Value=AEB_ObjectMovingState.Moving  SampleTime=inf
  Enumerated Constant18 : simulink/Sources/Enumerated Constant  OutDataTypeStr=Enum: AEB_ObjectMovingState  Value=AEB_ObjectMovingState.Still  SampleTime=inf
  Enumerated Constant19 : simulink/Sources/Enumerated Constant  OutDataTypeStr=Enum: AEB_ObjectMovingState  Value=AEB_ObjectMovingState.Stopped  SampleTime=inf
  Enumerated Constant20 : simulink/Sources/Enumerated Constant  OutDataTypeStr=Enum: AEB_ObjectMovingState  Value=AEB_ObjectMovingState.UnknownState  SampleTime=inf
  MovingStatus_Subsystem : Subsystem
  Multiport Switch3 : MultiPortSwitch  DataPortOrder=Specify indices  DataPortForDefault=Additional data port  DiagnosticForDefault=None
  ObjType_Subsystem1 : Subsystem
  FC_ObjectTypeFilt : Outport
  FC_ObjectMovingStateFilt : Outport  Port=2
  FC_ObjectMovingOrientationFilt : Outport  Port=3
  wires:
  Multiport Switch3/1 -> FC_ObjectMovingStateFilt/1
  Enumerated Constant19/1 -> Multiport Switch3/4
  Enumerated Constant13/1 -> Multiport Switch3/3
  Enumerated Constant18/1 -> Multiport Switch3/2
  MovingStatus_Subsystem/1(MovingStatusOut) -> Multiport Switch3/1
  Enumerated Constant20/1 -> Multiport Switch3/5
  ObjType_Subsystem1/1(ObjType) -> Data Type Conversion1/1  "ObjectType"
  FC_ObjectType/1 -> ObjType_Subsystem1/1(Alg_ObjType)
  Data Type Conversion1/1 -> FC_ObjectTypeFilt/1  "ObjectType"
  FC_ObjectMovingState/1 -> MovingStatus_Subsystem/1(Alg_MovingStatus)
  FC_ObjectMovingOrientation/1 -> Data Type Conversion/1
  Data Type Conversion/1 -> FC_ObjectMovingOrientationFilt/1  "ObjectMovingOrientation"

== system TosAeb/FcwAebJa_Step_sys/ALG_AEBSystem/ALG_AEBInput/VehCanInfoMap/DrivingModeDis_HCU_Map ==
  note: 0x0:Auto/Normal Mode started
      | 0x1:EV Mode started
      | 0x2:Save Mode started
      | 0x3:SPORT Mode started
      | 0x4:AWD Normal Mode started
      | 0x5:Snow Mode started
      | 0x6:Muddy Mode started
      | 0x7:Sand Mode started
      | 0x8:ECO
      | 0x9:SPORT Plus Mode started
      | 0xA-0xF:Reserved
  HCU_DrvMod : Inport
  Constant : Constant  Value=CNDrvModeEco
  Constant1 : Constant  Value=CNDrvModeNorm
  Constant2 : Constant  Value=CNDrvModeSport
  Constant3 : Constant  Value=CNDrvModeOther
  Multiport Switch2 : MultiPortSwitch  DataPortOrder=Specify indices  DataPortIndices={[1,2],[3,4],[5,6,7]}  DataPortForDefault=Additional data port  DiagnosticForDefault=None
  DrivingModeDis : Outport
  wires:
  Constant3/1 -> Multiport Switch2/5
  Constant2/1 -> Multiport Switch2/4
  Constant1/1 -> Multiport Switch2/3
  Constant/1 -> Multiport Switch2/2
  Multiport Switch2/1 -> DrivingModeDis/1
  HCU_DrvMod/1 -> Multiport Switch2/1

== system TosAeb/FcwAebJa_Step_sys/ALG_AEBSystem/ALG_AEBInput/VehCanInfoMap/FCTB_SigMap ==
  FCTB_ABA_Req : Inport
  FCTB_ABP_Req : Inport  Port=2
  FCTB_BrkgReq : Inport  Port=3
  Compare To Constant1 : simulink/Logic and Bit Operations/Compare To Constant  relop===  const=TRUE  OutDataTypeStr=boolean
  Compare To Constant2 : simulink/Logic and Bit Operations/Compare To Constant  relop===  const=TRUE  OutDataTypeStr=boolean
  Compare To Constant3 : simulink/Logic and Bit Operations/Compare To Constant  relop===  const=TRUE  OutDataTypeStr=boolean
  Enumerated Constant1 : simulink/Sources/Enumerated Constant  OutDataTypeStr=Enum: ModSts  Value=ModSts.StandbyState  SampleTime=inf
  Enumerated Constant2 : simulink/Sources/Enumerated Constant  OutDataTypeStr=Enum: ModSts  Value=ModSts.ActiveState  SampleTime=inf
  Enumerated Constant3 : simulink/Sources/Enumerated Constant  OutDataTypeStr=Enum: ModSts  Value=ModSts.StandbyState  SampleTime=inf
  Enumerated Constant4 : simulink/Sources/Enumerated Constant  OutDataTypeStr=Enum: ModSts  Value=ModSts.StandbyState  SampleTime=inf
  Enumerated Constant5 : simulink/Sources/Enumerated Constant  OutDataTypeStr=Enum: ModSts  Value=ModSts.ActiveState  SampleTime=inf
  Enumerated Constant6 : simulink/Sources/Enumerated Constant  OutDataTypeStr=Enum: ModSts  Value=ModSts.ActiveState  SampleTime=inf
  Switch1 : Switch  Criteria=u2 ~= 0
  Switch2 : Switch  Criteria=u2 ~= 0
  Switch3 : Switch  Criteria=u2 ~= 0
  FCTB_ABA_ReqFilt : Outport
  FCTB_ABP_ReqFilt : Outport  Port=2
  FCTB_BrkgReqFilt : Outport  Port=3
  wires:
  Switch3/1 -> FCTB_ABA_ReqFilt/1
  FCTB_ABA_Req/1 -> Compare To Constant2/1
  Switch1/1 -> FCTB_ABP_ReqFilt/1
  FCTB_ABP_Req/1 -> Compare To Constant1/1
  FCTB_BrkgReq/1 -> Compare To Constant3/1
  Switch2/1 -> FCTB_BrkgReqFilt/1
  Compare To Constant3/1 -> Switch2/2
  Enumerated Constant4/1 -> Switch2/3
  Enumerated Constant5/1 -> Switch2/1
  Enumerated Constant2/1 -> Switch1/1
  Enumerated Constant1/1 -> Switch1/3
  Compare To Constant1/1 -> Switch1/2
  Compare To Constant2/1 -> Switch3/2
  Enumerated Constant3/1 -> Switch3/3
  Enumerated Constant6/1 -> Switch3/1

== system TosAeb/FcwAebJa_Step_sys/ALG_AEBSystem/ALG_AEBInput/VehCanInfoMap/Subsystem ==
  ALG_LinghtStatus : Inport
  Constant3 : Constant  Value=0
  Constant4 : Constant
  Multiport Switch3 : MultiPortSwitch  DataPortOrder=Specify indices  DataPortIndices={1,2}  DataPortForDefault=Additional data port  DiagnosticForDefault=None
  LTurnLmpSwtSts : Outport
  wires:
  ALG_LinghtStatus/1 -> Multiport Switch3/1
  Constant3/1 -> Multiport Switch3/3, Multiport Switch3/4
  Multiport Switch3/1 -> LTurnLmpSwtSts/1  "LTurnLmpSwtSts"
  Constant4/1 -> Multiport Switch3/2

== system TosAeb/FcwAebJa_Step_sys/ALG_AEBSystem/ALG_AEBInput/VehCanInfoMap/Subsystem1 ==
  ALG_LinghtStatus : Inport
  Constant1 : Constant
  Constant3 : Constant  Value=0
  Constant4 : Constant  Value=0
  Multiport Switch3 : MultiPortSwitch  DataPortOrder=Specify indices  DataPortIndices={1,2}  DataPortForDefault=Additional data port  DiagnosticForDefault=None
  LTurnLmpSwtSts : Outport
  wires:
  Constant4/1 -> Multiport Switch3/2
  Multiport Switch3/1 -> LTurnLmpSwtSts/1  "RTurnLmpSwtSts"
  Constant3/1 -> Multiport Switch3/4
  ALG_LinghtStatus/1 -> Multiport Switch3/1
  Constant1/1 -> Multiport Switch3/3

== system TosAeb/FcwAebJa_Step_sys/ALG_AEBSystem/ALG_AEBInput/VehCanInfoMap/Subsystem2 ==
  ALG_AEBSwitchReq : Inport
  Constant1 : Constant
  Constant3 : Constant  Value=0
  Constant4 : Constant  Value=0
  Multiport Switch3 : MultiPortSwitch  DataPortOrder=Specify indices  DataPortIndices={1,2}  DataPortForDefault=Additional data port  DiagnosticForDefault=None
  AEBSwtSts : Outport
  wires:
  Constant4/1 -> Multiport Switch3/2
  Multiport Switch3/1 -> AEBSwtSts/1  "AEBSwtSts"
  Constant3/1 -> Multiport Switch3/4
  ALG_AEBSwitchReq/1 -> Multiport Switch3/1
  Constant1/1 -> Multiport Switch3/3

== system TosAeb/FcwAebJa_Step_sys/ALG_AEBSystem/ALG_AEBOutput/AEBS_BrkInfoCalc/AEBS_BrkInfoMap ==
  ABALvl : Inport
  ABATrigger : Inport  Port=2
  AEBTrigger : Inport  Port=3
  axTgtReqValue : Inport  Port=4
  acuteWarningTrigger : Inport  Port=5
  acuteWarningLvl : Inport  Port=6
  ABPTrigger : Inport  Port=7
  EBA_TgtDecel_Req : Inport  Port=8
  AEB_VehHldReq : Inport  Port=9
  AEB_BrkReqFunc : Inport  Port=10
  Bus Creator : BusCreator  Inputs=10  OutDataTypeStr=Bus: AEB_BRK_INFO_BUS  NonVirtualBus=on
      settings: InheritFromInputs=off
  Compare To Constant : simulink/Logic and Bit Operations/Compare To Constant  relop===  const=uint8(0)  OutDataTypeStr=boolean
  Compare To Constant1 : simulink/Logic and Bit Operations/Compare To Constant  relop=~=  const=uint8(0)  OutDataTypeStr=boolean
  Constant1 : Constant  Value=0  OutDataTypeStr=single
  Constant2 : Constant  Value=P_AEB_AWB_ConfigSet
  Constant3 : Constant  Value=P_AEB_AWB_ConfigSet
  Logical Operator : Logic  AllPortsSameDT=off  OutDataTypeStr=boolean
  Signal Conversion{,1..9} (10 alike) : SignalConversion  OverrideOpt=off
  Switch : Switch  Criteria=u2 ~= 0
  Switch1 : Switch  Criteria=u2 ~= 0
  AEB_BRK_INFO : Outport  OutDataTypeStr=Bus: AEB_BRK_INFO_BUS
  wires:
  Constant1/1 -> Switch1/3
  axTgtReqValue/1 -> Switch1/1
  Compare To Constant1/1 -> Logical Operator/2, Switch1/2
  Constant3/1 -> Compare To Constant1/1
  Constant2/1 -> Compare To Constant/1  "AEB_AWB_ConfigSet"
  acuteWarningTrigger/1 -> Switch/3
  AEBTrigger/1 -> Logical Operator/1, Switch/1
  Compare To Constant/1 -> Switch/2
  Switch1/1 -> Signal Conversion3/1
  Logical Operator/1 -> Signal Conversion2/1
  Bus Creator/1 -> AEB_BRK_INFO/1
  Signal Conversion9/1 -> Bus Creator/10  "AEB_VehHldReq"
  Signal Conversion8/1 -> Bus Creator/8  "AEB_BrkReqFunc"
  Signal Conversion7/1 -> Bus Creator/9  "EBA_TgtDecel_Req"
  AEB_VehHldReq/1 -> Signal Conversion9/1
  AEB_BrkReqFunc/1 -> Signal Conversion8/1
  EBA_TgtDecel_Req/1 -> Signal Conversion7/1
  ABPTrigger/1 -> Signal Conversion6/1
  acuteWarningLvl/1 -> Signal Conversion5/1
  ABALvl/1 -> Signal Conversion/1
  ABATrigger/1 -> Signal Conversion1/1
  Signal Conversion4/1 -> Bus Creator/4  "AEB_AWB_Req"
  Signal Conversion5/1 -> Bus Creator/5  "AEB_AWB_Level"
  Signal Conversion/1 -> Bus Creator/3  "AEB_ABA_Level"
  Switch/1 -> Signal Conversion4/1
  Signal Conversion3/1 -> Bus Creator/7  "AEB_TgtDecel_ReqValue"
  Signal Conversion2/1 -> Bus Creator/6  "AEB_TgtDecel_Req"
  Signal Conversion6/1 -> Bus Creator/1  "AEB_ABP_Req"
  Signal Conversion1/1 -> Bus Creator/2  "AEB_ABA_Req"

== system TosAeb/FcwAebJa_Step_sys/ALG_AEBSystem/ALG_AEBOutput/AEBS_BrkInfoCalc/AEB_BrkReqFuncCalc ==
  note: 0x0:No Request
      | 0x1:AEB-V
      | 0x2:AEB-VRU
      | 0x3:AEB-JA
      | 0x4:Reserved
      | 0x5:AEB-FCTB
      | 0x6-0x7:Reserved
  AEBpartFuncImpArb : Inport
  AEBfullFuncImpArb : Inport  Port=2
  EBAFuncImpArb : Inport  Port=3
  cycAEBpartFuncImpArb : Inport  Port=4
  cycAEBfullFuncImpArb : Inport  Port=5
  pedAEBfullFuncImpArb : Inport  Port=6
  JA_AEBfullFuncImpArb : Inport  Port=7
  JA_pedAEBRawModSts : Inport  Port=8
  FCTBFuncImpArb : Inport  Port=9
  Inter_AEB_BrkReqFuncFlow : Stateflow chart  TreatAsAtomicUnit=on
  AEB_BrkReqFunc : Outport
  wires:
  JA_AEBfullFuncImpArb/1 -> Inter_AEB_BrkReqFuncFlow/7(JA_AEBfullFuncImpArb)
  cycAEBfullFuncImpArb/1 -> Inter_AEB_BrkReqFuncFlow/5(cycAEBfullFuncImpArb)
  EBAFuncImpArb/1 -> Inter_AEB_BrkReqFuncFlow/3(EBAFuncImpArb)
  cycAEBpartFuncImpArb/1 -> Inter_AEB_BrkReqFuncFlow/4(cycAEBpartFuncImpArb)
  AEBpartFuncImpArb/1 -> Inter_AEB_BrkReqFuncFlow/1(AEBpartFuncImpArb)
  JA_pedAEBRawModSts/1 -> Inter_AEB_BrkReqFuncFlow/8(JA_pedAEBRawModSts)
  pedAEBfullFuncImpArb/1 -> Inter_AEB_BrkReqFuncFlow/6(pedAEBfullFuncImpArb)
  AEBfullFuncImpArb/1 -> Inter_AEB_BrkReqFuncFlow/2(AEBfullFuncImpArb)
  FCTBFuncImpArb/1 -> Inter_AEB_BrkReqFuncFlow/9(FCTBFuncImpArb)
  Inter_AEB_BrkReqFuncFlow/1(AEB_BrkReqFunc) -> AEB_BrkReqFunc/1

== system TosAeb/FcwAebJa_Step_sys/ALG_AEBSystem/ALG_AEBOutput/AEBS_BrkInfoCalc/AEB_VehHldReqCalc ==
  vehAEBpartRawModSts : Inport
  vehAEBfullRawModSts : Inport  Port=2
  vehEBARawModSts : Inport  Port=3
  pedAEBfullRawModSts : Inport  Port=4
  cycAEBpartRawModSts : Inport  Port=5
  cycAEBfullRawModSts : Inport  Port=6
  JA_vehAEBRawModSts : Inport  Port=7
  JA_pedAEBRawModSts : Inport  Port=8
  AEBtrigger : Inport  Port=9
  JA_cycAEBRawModSts : Inport  Port=10
  Compare To Constant : simulink/Logic and Bit Operations/Compare To Constant  relop===  const=TRUE  OutDataTypeStr=boolean
  Compare To Constant{1..9} (9 alike) : simulink/Logic and Bit Operations/Compare To Constant  relop===  const=ModSts.StandstillActiveState  OutDataTypeStr=boolean
  Logical Operator : Logic  AllPortsSameDT=off  OutDataTypeStr=boolean
  Logical Operator1 : Logic  Operator=OR  Inputs=9  AllPortsSameDT=off  OutDataTypeStr=boolean
  Inter_AEB_VehHldReq : Outport
  wires:
  Compare To Constant8/1 -> Logical Operator1/8
  JA_pedAEBRawModSts/1 -> Compare To Constant8/1
  Compare To Constant7/1 -> Logical Operator1/7
  Compare To Constant6/1 -> Logical Operator1/6
  Compare To Constant5/1 -> Logical Operator1/5
  Compare To Constant4/1 -> Logical Operator1/4
  Compare To Constant3/1 -> Logical Operator1/3
  Compare To Constant1/1 -> Logical Operator1/2
  Compare To Constant2/1 -> Logical Operator1/1
  JA_vehAEBRawModSts/1 -> Compare To Constant7/1
  cycAEBfullRawModSts/1 -> Compare To Constant6/1
  cycAEBpartRawModSts/1 -> Compare To Constant5/1
  pedAEBfullRawModSts/1 -> Compare To Constant4/1
  vehEBARawModSts/1 -> Compare To Constant3/1
  vehAEBfullRawModSts/1 -> Compare To Constant1/1
  vehAEBpartRawModSts/1 -> Compare To Constant2/1
  Logical Operator/1 -> Inter_AEB_VehHldReq/1
  Logical Operator1/1 -> Logical Operator/2
  Compare To Constant/1 -> Logical Operator/1
  AEBtrigger/1 -> Compare To Constant/1
  JA_cycAEBRawModSts/1 -> Compare To Constant9/1
  Compare To Constant9/1 -> Logical Operator1/9

== system TosAeb/FcwAebJa_Step_sys/ALG_AEBSystem/ALG_AEBOutput/AEBS_BrkInfoCalc/EBA_TgtDecel_ReqJudge ==
  AEBtrigger : Inport
  EBAFuncImpArb : Inport  Port=2
  Compare To Constant : simulink/Logic and Bit Operations/Compare To Constant  relop===  const=ModSts.ActiveState  OutDataTypeStr=boolean
  Compare To Constant1 : simulink/Logic and Bit Operations/Compare To Constant  relop===  const=TRUE  OutDataTypeStr=boolean
  Logical Operator : Logic  AllPortsSameDT=off  OutDataTypeStr=boolean
  Inter_EBA_TgtDecel_Req : Outport
  wires:
  Compare To Constant1/1 -> Logical Operator/1
  Compare To Constant/1 -> Logical Operator/2
  AEBtrigger/1 -> Compare To Constant1/1
  Logical Operator/1 -> Inter_EBA_TgtDecel_Req/1
  EBAFuncImpArb/1 -> Compare To Constant/1
